simulink model "CDF_VSVPWM_Grid_Parallel"  (R2024b)
settings: Solver=VariableStepAuto  StartTime=0.0  StopTime=0.1  FixedStep=1e-7  MaxStep=1e-5  RelTol=1e-4
InitFcn:
  | Ts=1/10e3;
  | Udc=600;
  | C=4000e-6;
  | m=0.7;
libraries: simulink x24, spsMosfetLib x24, spsSeriesRLCBranchLib x16, spsDiodeLib x12, spsabctoAlphaBetaZeroLib x5, spsThreePhaseVIMeasurementLib x4, spsVoltageMeasurementLib x4, spsAlphaBetaZerotoabcLib x2, spsStairGeneratorLib x2, spsOnDelayLib x2, spsdq0toAlphaBetaZeroLib x2, spsBreakerLib x1
(part 3 of 5)

== system CDF_VSVPWM_Grid_Parallel/VSVPWM/生成时间调制波/Ⅴ扇区的调制信号计算/1-4 ==
  time : Inport
  Demux : Demux  Outputs=5
  MATLAB Function : MATLAB Function  TreatAsAtomicUnit=on
  MATLAB Function1 : MATLAB Function  TreatAsAtomicUnit=on
  MATLAB Function2 : MATLAB Function  TreatAsAtomicUnit=on
  Mux : Mux  Inputs=6
  Tabc12 : Outport
  wires:
  time/1 -> Demux/1
  Demux/3 -> MATLAB Function2/3(T3), MATLAB Function1/3(unused), MATLAB Function/3(T3)
  Mux/1 -> Tabc12/1
  Demux/1 -> MATLAB Function2/1(unused), MATLAB Function1/1(T1), MATLAB Function/1(T1)
  Demux/2 -> MATLAB Function2/2(T2), MATLAB Function1/2(T2), MATLAB Function/2(T2)
  Demux/4 -> MATLAB Function2/4(T4), MATLAB Function1/4(unused1), MATLAB Function/4(unused)
  Demux/5 -> MATLAB Function2/5(T5), MATLAB Function1/5(T5), MATLAB Function/5(unused1)
  MATLAB Function/1(ta1) -> Mux/5
  MATLAB Function/2(ta2) -> Mux/6
  MATLAB Function1/1(tb1) -> Mux/1
  MATLAB Function1/2(tb2) -> Mux/2
  MATLAB Function2/1(tc1) -> Mux/3
  MATLAB Function2/2(tc2) -> Mux/4

== system CDF_VSVPWM_Grid_Parallel/VSVPWM/生成时间调制波/Ⅴ扇区的调制信号计算/1-5 ==
  time : Inport
  Demux : Demux  Outputs=5
  MATLAB Function : MATLAB Function  TreatAsAtomicUnit=on
  MATLAB Function1 : MATLAB Function  TreatAsAtomicUnit=on
  MATLAB Function2 : MATLAB Function  TreatAsAtomicUnit=on
  Mux : Mux  Inputs=6
  Tabc12 : Outport
  wires:
  Demux/5 -> MATLAB Function/5(unused), MATLAB Function1/5(T5), MATLAB Function2/5(T5)
  Demux/4 -> MATLAB Function/4(T4), MATLAB Function1/4(T4), MATLAB Function2/4(T4)
  Demux/2 -> MATLAB Function/2(T2), MATLAB Function1/2(T2), MATLAB Function2/2(T2)
  Demux/1 -> MATLAB Function/1(T1), MATLAB Function1/1(T1), MATLAB Function2/1(unused)
  Demux/3 -> MATLAB Function/3(T3), MATLAB Function1/3(unused), MATLAB Function2/3(T3)
  time/1 -> Demux/1
  MATLAB Function1/2(tb2) -> Mux/2
  MATLAB Function2/1(tc1) -> Mux/3
  Mux/1 -> Tabc12/1
  MATLAB Function/1(ta1) -> Mux/5
  MATLAB Function2/2(tc2) -> Mux/4
  MATLAB Function/2(ta2) -> Mux/6
  MATLAB Function1/1(tb1) -> Mux/1

== system CDF_VSVPWM_Grid_Parallel/VSVPWM/生成时间调制波/Ⅵ扇区的调制信号计算/2-1 ==
  time : Inport
  Demux : Demux  Outputs=5
  MATLAB Function : MATLAB Function  TreatAsAtomicUnit=on
  MATLAB Function1 : MATLAB Function  TreatAsAtomicUnit=on
  MATLAB Function2 : MATLAB Function  TreatAsAtomicUnit=on
  Mux : Mux  Inputs=6
  Tabc12 : Outport
  wires:
  time/1 -> Demux/1
  Demux/3 -> MATLAB Function2/3(unused2), MATLAB Function1/3(unused), MATLAB Function/3(unused1)
  Demux/1 -> MATLAB Function2/1(unused), MATLAB Function1/1(T1), MATLAB Function/1(T1)
  Demux/2 -> MATLAB Function2/2(unused1), MATLAB Function1/2(T2), MATLAB Function/2(unused)
  Demux/4 -> MATLAB Function2/4(T4), MATLAB Function1/4(unused1), MATLAB Function/4(unused2)
  Demux/5 -> MATLAB Function2/5(T5), MATLAB Function1/5(unused2), MATLAB Function/5(T5)
  MATLAB Function1/2(tb2) -> Mux/2
  MATLAB Function1/1(tb1) -> Mux/1
  MATLAB Function2/2(tc2) -> Mux/4
  MATLAB Function2/1(tc1) -> Mux/3
  MATLAB Function/2(ta2) -> Mux/6
  MATLAB Function/1(ta1) -> Mux/5
  Mux/1 -> Tabc12/1

== system CDF_VSVPWM_Grid_Parallel/VSVPWM/生成时间调制波/Ⅵ扇区的调制信号计算/2-2 ==
  time : Inport
  Demux : Demux  Outputs=5
  MATLAB Function : MATLAB Function  TreatAsAtomicUnit=on
  MATLAB Function1 : MATLAB Function  TreatAsAtomicUnit=on
  MATLAB Function2 : MATLAB Function  TreatAsAtomicUnit=on
  Mux : Mux  Inputs=6
  Tabc12 : Outport
  wires:
  Demux/5 -> MATLAB Function/5(T5), MATLAB Function1/5(unused1), MATLAB Function2/5(T5)
  Demux/4 -> MATLAB Function/4(unused2), MATLAB Function1/4(unused), MATLAB Function2/4(T4)
  Demux/2 -> MATLAB Function/2(unused), MATLAB Function1/2(T2), MATLAB Function2/2(unused1)
  Demux/1 -> MATLAB Function/1(T1), MATLAB Function1/1(T1), MATLAB Function2/1(unused)
  Demux/3 -> MATLAB Function/3(unused1), MATLAB Function1/3(T3), MATLAB Function2/3(T3)
  time/1 -> Demux/1
  MATLAB Function1/2(tb2) -> Mux/2
  MATLAB Function1/1(tb1) -> Mux/1
  MATLAB Function2/2(tc2) -> Mux/4
  MATLAB Function2/1(tc1) -> Mux/3
  MATLAB Function/2(ta2) -> Mux/6
  MATLAB Function/1(ta1) -> Mux/5
  Mux/1 -> Tabc12/1

== system CDF_VSVPWM_Grid_Parallel/VSVPWM/生成时间调制波/Ⅵ扇区的调制信号计算/2-3 ==
  time : Inport
  Demux : Demux  Outputs=5
  MATLAB Function : MATLAB Function  TreatAsAtomicUnit=on
  MATLAB Function1 : MATLAB Function  TreatAsAtomicUnit=on
  MATLAB Function2 : MATLAB Function  TreatAsAtomicUnit=on
  Mux : Mux  Inputs=6
  Tabc12 : Outport
  wires:
  time/1 -> Demux/1
  Demux/3 -> MATLAB Function2/3(T3), MATLAB Function1/3(T3), MATLAB Function/3(unused)
  Demux/1 -> MATLAB Function2/1(unused), MATLAB Function1/1(T1), MATLAB Function/1(T1)
  Demux/2 -> MATLAB Function2/2(T2), MATLAB Function1/2(T2), MATLAB Function/2(T2)
  Demux/4 -> MATLAB Function2/4(T4), MATLAB Function1/4(unused), MATLAB Function/4(unused1)
  Demux/5 -> MATLAB Function2/5(T5), MATLAB Function1/5(unused1), MATLAB Function/5(T5)
  MATLAB Function1/2(tb2) -> Mux/2
  MATLAB Function1/1(tb1) -> Mux/1
  MATLAB Function2/2(tc2) -> Mux/4
  MATLAB Function2/1(tc1) -> Mux/3
  MATLAB Function/2(ta2) -> Mux/6
  MATLAB Function/1(ta1) -> Mux/5
  Mux/1 -> Tabc12/1

== system CDF_VSVPWM_Grid_Parallel/VSVPWM/生成时间调制波/Ⅵ扇区的调制信号计算/2-4 ==
  time : Inport
  Demux : Demux  Outputs=5
  MATLAB Function : MATLAB Function  TreatAsAtomicUnit=on
  MATLAB Function1 : MATLAB Function  TreatAsAtomicUnit=on
  MATLAB Function2 : MATLAB Function  TreatAsAtomicUnit=on
  Mux : Mux  Inputs=6
  Tabc12 : Outport
  wires:
  Demux/5 -> MATLAB Function/5(T5), MATLAB Function1/5(unused), MATLAB Function2/5(T5)
  Demux/4 -> MATLAB Function/4(T4), MATLAB Function1/4(T4), MATLAB Function2/4(T4)
  Demux/2 -> MATLAB Function/2(unused), MATLAB Function1/2(T2), MATLAB Function2/2(unused1)
  Demux/1 -> MATLAB Function/1(T1), MATLAB Function1/1(T1), MATLAB Function2/1(unused)
  Demux/3 -> MATLAB Function/3(unused1), MATLAB Function1/3(T3), MATLAB Function2/3(T3)
  time/1 -> Demux/1
  MATLAB Function1/2(tb2) -> Mux/2
  MATLAB Function1/1(tb1) -> Mux/1
  MATLAB Function2/2(tc2) -> Mux/4
  MATLAB Function2/1(tc1) -> Mux/3
  MATLAB Function/2(ta2) -> Mux/6
  MATLAB Function/1(ta1) -> Mux/5
  Mux/1 -> Tabc12/1

== system CDF_VSVPWM_Grid_Parallel/VSVPWM/生成时间调制波/Ⅵ扇区的调制信号计算/2-5 ==
  time : Inport
  Demux : Demux  Outputs=5
  MATLAB Function : MATLAB Function  TreatAsAtomicUnit=on
  MATLAB Function1 : MATLAB Function  TreatAsAtomicUnit=on
  MATLAB Function2 : MATLAB Function  TreatAsAtomicUnit=on
  Mux : Mux  Inputs=6
  Tabc12 : Outport
  wires:
  time/1 -> Demux/1
  Demux/3 -> MATLAB Function2/3(T3), MATLAB Function1/3(T3), MATLAB Function/3(unused)
  Demux/1 -> MATLAB Function2/1(unused), MATLAB Function1/1(T1), MATLAB Function/1(T1)
  Demux/2 -> MATLAB Function2/2(T2), MATLAB Function1/2(T2), MATLAB Function/2(T2)
  Demux/4 -> MATLAB Function2/4(T4), MATLAB Function1/4(T4), MATLAB Function/4(T4)
  Demux/5 -> MATLAB Function2/5(T5), MATLAB Function1/5(unused), MATLAB Function/5(T5)
  MATLAB Function1/2(tb2) -> Mux/2
  MATLAB Function1/1(tb1) -> Mux/1
  MATLAB Function2/2(tc2) -> Mux/4
  MATLAB Function2/1(tc1) -> Mux/3
  MATLAB Function/2(ta2) -> Mux/6
  MATLAB Function/1(ta1) -> Mux/5
  Mux/1 -> Tabc12/1

== system CDF_VSVPWM_Grid_Parallel/VSVPWM1/生成时间调制波/Ⅰ扇区的调制信号计算/1-1 ==
  time : Inport
  Demux : Demux  Outputs=5
  MATLAB Function : MATLAB Function  TreatAsAtomicUnit=on
  MATLAB Function1 : MATLAB Function  TreatAsAtomicUnit=on
  MATLAB Function2 : MATLAB Function  TreatAsAtomicUnit=on
  Mux : Mux  Inputs=6
  Scope : Scope
  Tabc12 : Outport
  wires:
  MATLAB Function/1(ta1) -> Mux/1
  time/1 -> Demux/1
  Mux/1 -> Scope/1, Tabc12/1
  Demux/1 -> MATLAB Function2/1(unused), MATLAB Function1/1(T1), MATLAB Function/1(T1)
  MATLAB Function/2(ta2) -> Mux/2
  MATLAB Function1/1(tb1) -> Mux/3
  MATLAB Function1/2(tb2) -> Mux/4
  MATLAB Function2/1(tc1) -> Mux/5
  MATLAB Function2/2(tc2) -> Mux/6
  Demux/2 -> MATLAB Function2/2(unused1), MATLAB Function1/2(unused), MATLAB Function/2(T2)
  Demux/3 -> MATLAB Function2/3(unused2), MATLAB Function1/3(unused1), MATLAB Function/3(unused)
  Demux/4 -> MATLAB Function2/4(T4), MATLAB Function1/4(unused2), MATLAB Function/4(unused1)
  Demux/5 -> MATLAB Function2/5(T5), MATLAB Function1/5(T5), MATLAB Function/5(unused2)

== system CDF_VSVPWM_Grid_Parallel/VSVPWM1/生成时间调制波/Ⅰ扇区的调制信号计算/1-2 ==
  time : Inport
  Demux : Demux  Outputs=5
  MATLAB Function : MATLAB Function  TreatAsAtomicUnit=on
  MATLAB Function1 : MATLAB Function  TreatAsAtomicUnit=on
  MATLAB Function2 : MATLAB Function  TreatAsAtomicUnit=on
  Mux : Mux  Inputs=6
  Tabc12 : Outport
  wires:
  MATLAB Function2/2(tc2) -> Mux/6
  MATLAB Function2/1(tc1) -> Mux/5
  MATLAB Function1/2(tb2) -> Mux/4
  MATLAB Function1/1(tb1) -> Mux/3
  MATLAB Function/2(ta2) -> Mux/2
  Demux/5 -> MATLAB Function/5(unused1), MATLAB Function1/5(T5), MATLAB Function2/5(T5)
  Demux/4 -> MATLAB Function/4(unused), MATLAB Function1/4(unused2), MATLAB Function2/4(T4)
  Demux/2 -> MATLAB Function/2(T2), MATLAB Function1/2(unused), MATLAB Function2/2(unused1)
  Demux/1 -> MATLAB Function/1(T1), MATLAB Function1/1(T1), MATLAB Function2/1(unused)
  Mux/1 -> Tabc12/1
  Demux/3 -> MATLAB Function/3(T3), MATLAB Function1/3(unused1), MATLAB Function2/3(T3)
  time/1 -> Demux/1
  MATLAB Function/1(ta1) -> Mux/1

== system CDF_VSVPWM_Grid_Parallel/VSVPWM1/生成时间调制波/Ⅰ扇区的调制信号计算/1-3 ==
  time : Inport
  Demux : Demux  Outputs=5
  MATLAB Function : MATLAB Function  TreatAsAtomicUnit=on
  MATLAB Function1 : MATLAB Function  TreatAsAtomicUnit=on
  MATLAB Function2 : MATLAB Function  TreatAsAtomicUnit=on
  Mux : Mux  Inputs=6
  Tabc12 : Outport
  wires:
  MATLAB Function/1(ta1) -> Mux/1
  time/1 -> Demux/1
  Demux/3 -> MATLAB Function2/3(T3), MATLAB Function1/3(unused1), MATLAB Function/3(T3)
  Mux/1 -> Tabc12/1
  Demux/1 -> MATLAB Function2/1(unused), MATLAB Function1/1(T1), MATLAB Function/1(T1)
  Demux/2 -> MATLAB Function2/2(unused1), MATLAB Function1/2(unused), MATLAB Function/2(T2)
  Demux/4 -> MATLAB Function2/4(T4), MATLAB Function1/4(T4), MATLAB Function/4(T4)
  Demux/5 -> MATLAB Function2/5(T5), MATLAB Function1/5(T5), MATLAB Function/5(unused)
  MATLAB Function/2(ta2) -> Mux/2
  MATLAB Function1/1(tb1) -> Mux/3
  MATLAB Function1/2(tb2) -> Mux/4
  MATLAB Function2/1(tc1) -> Mux/5
  MATLAB Function2/2(tc2) -> Mux/6

== system CDF_VSVPWM_Grid_Parallel/VSVPWM1/生成时间调制波/Ⅰ扇区的调制信号计算/1-4 ==
  time : Inport
  Demux : Demux  Outputs=5
  MATLAB Function : MATLAB Function  TreatAsAtomicUnit=on
  MATLAB Function1 : MATLAB Function  TreatAsAtomicUnit=on
  MATLAB Function2 : MATLAB Function  TreatAsAtomicUnit=on
  Mux : Mux  Inputs=6
  Tabc12 : Outport
  wires:
  MATLAB Function2/2(tc2) -> Mux/6
  MATLAB Function2/1(tc1) -> Mux/5
  MATLAB Function1/2(tb2) -> Mux/4
  MATLAB Function1/1(tb1) -> Mux/3
  MATLAB Function/2(ta2) -> Mux/2
  Demux/5 -> MATLAB Function/5(unused1), MATLAB Function1/5(T5), MATLAB Function2/5(T5)
  Demux/4 -> MATLAB Function/4(unused), MATLAB Function1/4(unused1), MATLAB Function2/4(T4)
  Demux/2 -> MATLAB Function/2(T2), MATLAB Function1/2(T2), MATLAB Function2/2(T2)
  Demux/1 -> MATLAB Function/1(T1), MATLAB Function1/1(T1), MATLAB Function2/1(unused)
  Mux/1 -> Tabc12/1
  Demux/3 -> MATLAB Function/3(T3), MATLAB Function1/3(unused), MATLAB Function2/3(T3)
  time/1 -> Demux/1
  MATLAB Function/1(ta1) -> Mux/1

== system CDF_VSVPWM_Grid_Parallel/VSVPWM1/生成时间调制波/Ⅰ扇区的调制信号计算/1-5 ==
  time : Inport
  Demux : Demux  Outputs=5
  MATLAB Function : MATLAB Function  TreatAsAtomicUnit=on
  MATLAB Function1 : MATLAB Function  TreatAsAtomicUnit=on
  MATLAB Function2 : MATLAB Function  TreatAsAtomicUnit=on
  Mux : Mux  Inputs=6
  Tabc12 : Outport
  wires:
  MATLAB Function/1(ta1) -> Mux/1
  time/1 -> Demux/1
  Demux/3 -> MATLAB Function2/3(T3), MATLAB Function1/3(unused), MATLAB Function/3(T3)
  Mux/1 -> Tabc12/1
  Demux/1 -> MATLAB Function2/1(unused), MATLAB Function1/1(T1), MATLAB Function/1(T1)
  Demux/2 -> MATLAB Function2/2(T2), MATLAB Function1/2(T2), MATLAB Function/2(T2)
  Demux/4 -> MATLAB Function2/4(T4), MATLAB Function1/4(T4), MATLAB Function/4(T4)
  Demux/5 -> MATLAB Function2/5(T5), MATLAB Function1/5(T5), MATLAB Function/5(unused)
  MATLAB Function/2(ta2) -> Mux/2
  MATLAB Function1/1(tb1) -> Mux/3
  MATLAB Function1/2(tb2) -> Mux/4
  MATLAB Function2/1(tc1) -> Mux/5
  MATLAB Function2/2(tc2) -> Mux/6

== system CDF_VSVPWM_Grid_Parallel/VSVPWM1/生成时间调制波/Ⅱ扇区的调制信号计算/2-1 ==
  time : Inport
  Demux : Demux  Outputs=5
  MATLAB Function : MATLAB Function  TreatAsAtomicUnit=on
  MATLAB Function1 : MATLAB Function  TreatAsAtomicUnit=on
  MATLAB Function2 : MATLAB Function  TreatAsAtomicUnit=on
  Mux : Mux  Inputs=6
  Scope : Scope  NumInputPorts=6
  Scope1 : Scope
  Tabc12 : Outport
  wires:
  MATLAB Function2/2(tc2) -> Scope/6, Mux/6
  MATLAB Function2/1(tc1) -> Scope/5, Mux/5
  MATLAB Function1/2(tb2) -> Scope/4, Mux/4
  MATLAB Function1/1(tb1) -> Scope/3, Mux/3
  MATLAB Function/2(ta2) -> Mux/2, Scope/2
  Demux/5 -> MATLAB Function/5(T5), MATLAB Function1/5(unused2), MATLAB Function2/5(T5)
  Demux/4 -> MATLAB Function/4(unused2), MATLAB Function1/4(unused1), MATLAB Function2/4(T4)
  Demux/2 -> MATLAB Function/2(unused), MATLAB Function1/2(T2), MATLAB Function2/2(unused1)
  Demux/1 -> MATLAB Function/1(T1), MATLAB Function1/1(T1), MATLAB Function2/1(unused)
  Mux/1 -> Scope1/1, Tabc12/1
  Demux/3 -> MATLAB Function/3(unused1), MATLAB Function1/3(unused), MATLAB Function2/3(unused2)
  time/1 -> Demux/1
  MATLAB Function/1(ta1) -> Scope/1, Mux/1

== system CDF_VSVPWM_Grid_Parallel/VSVPWM1/生成时间调制波/Ⅱ扇区的调制信号计算/2-2 ==
  time : Inport
  Demux : Demux  Outputs=5
  MATLAB Function : MATLAB Function  TreatAsAtomicUnit=on
  MATLAB Function1 : MATLAB Function  TreatAsAtomicUnit=on
  MATLAB Function2 : MATLAB Function  TreatAsAtomicUnit=on
  Mux : Mux  Inputs=6
  Tabc12 : Outport
  wires:
  MATLAB Function/1(ta1) -> Mux/1
  time/1 -> Demux/1
  Demux/3 -> MATLAB Function2/3(T3), MATLAB Function1/3(T3), MATLAB Function/3(unused1)
  Mux/1 -> Tabc12/1
  Demux/1 -> MATLAB Function2/1(unused), MATLAB Function1/1(T1), MATLAB Function/1(T1)
  Demux/2 -> MATLAB Function2/2(unused1), MATLAB Function1/2(T2), MATLAB Function/2(unused)
  Demux/4 -> MATLAB Function2/4(T4), MATLAB Function1/4(unused), MATLAB Function/4(unused2)
  Demux/5 -> MATLAB Function2/5(T5), MATLAB Function1/5(unused1), MATLAB Function/5(T5)
  MATLAB Function/2(ta2) -> Mux/2
  MATLAB Function1/1(tb1) -> Mux/3
  MATLAB Function1/2(tb2) -> Mux/4
  MATLAB Function2/1(tc1) -> Mux/5
  MATLAB Function2/2(tc2) -> Mux/6

== system CDF_VSVPWM_Grid_Parallel/VSVPWM1/生成时间调制波/Ⅱ扇区的调制信号计算/2-3 ==
  time : Inport
  Demux : Demux  Outputs=5
  MATLAB Function : MATLAB Function  TreatAsAtomicUnit=on
  MATLAB Function1 : MATLAB Function  TreatAsAtomicUnit=on
  MATLAB Function2 : MATLAB Function  TreatAsAtomicUnit=on
  Mux : Mux  Inputs=6
  Tabc12 : Outport
  wires:
  MATLAB Function2/2(tc2) -> Mux/6
  MATLAB Function2/1(tc1) -> Mux/5
  MATLAB Function1/2(tb2) -> Mux/4
  MATLAB Function1/1(tb1) -> Mux/3
  MATLAB Function/2(ta2) -> Mux/2
  Demux/5 -> MATLAB Function/5(T5), MATLAB Function1/5(unused1), MATLAB Function2/5(T5)
  Demux/4 -> MATLAB Function/4(unused1), MATLAB Function1/4(unused), MATLAB Function2/4(T4)
  Demux/2 -> MATLAB Function/2(T2), MATLAB Function1/2(T2), MATLAB Function2/2(T2)
  Demux/1 -> MATLAB Function/1(T1), MATLAB Function1/1(T1), MATLAB Function2/1(unused)
  Mux/1 -> Tabc12/1
  Demux/3 -> MATLAB Function/3(unused), MATLAB Function1/3(T3), MATLAB Function2/3(T3)
  time/1 -> Demux/1
  MATLAB Function/1(ta1) -> Mux/1

== system CDF_VSVPWM_Grid_Parallel/VSVPWM1/生成时间调制波/Ⅱ扇区的调制信号计算/2-4 ==
  time : Inport
  Demux : Demux  Outputs=5
  MATLAB Function : MATLAB Function  TreatAsAtomicUnit=on
  MATLAB Function1 : MATLAB Function  TreatAsAtomicUnit=on
  MATLAB Function2 : MATLAB Function  TreatAsAtomicUnit=on
  Mux : Mux  Inputs=6
  Tabc12 : Outport
  wires:
  MATLAB Function/1(ta1) -> Mux/1
  time/1 -> Demux/1
  Demux/3 -> MATLAB Function2/3(T3), MATLAB Function1/3(T3), MATLAB Function/3(unused1)
  Mux/1 -> Tabc12/1
  Demux/1 -> MATLAB Function2/1(unused), MATLAB Function1/1(T1), MATLAB Function/1(T1)
  Demux/2 -> MATLAB Function2/2(unused1), MATLAB Function1/2(T2), MATLAB Function/2(unused)
  Demux/4 -> MATLAB Function2/4(T4), MATLAB Function1/4(T4), MATLAB Function/4(T4)
  Demux/5 -> MATLAB Function2/5(T5), MATLAB Function1/5(unused), MATLAB Function/5(T5)
  MATLAB Function/2(ta2) -> Mux/2
  MATLAB Function1/1(tb1) -> Mux/3
  MATLAB Function1/2(tb2) -> Mux/4
  MATLAB Function2/1(tc1) -> Mux/5
  MATLAB Function2/2(tc2) -> Mux/6

== system CDF_VSVPWM_Grid_Parallel/VSVPWM1/生成时间调制波/Ⅱ扇区的调制信号计算/2-5 ==
  time : Inport
  Demux : Demux  Outputs=5
  MATLAB Function : MATLAB Function  TreatAsAtomicUnit=on
  MATLAB Function1 : MATLAB Function  TreatAsAtomicUnit=on
  MATLAB Function2 : MATLAB Function  TreatAsAtomicUnit=on
  Mux : Mux  Inputs=6
  Tabc12 : Outport
  wires:
  MATLAB Function2/2(tc2) -> Mux/6
  MATLAB Function2/1(tc1) -> Mux/5
  MATLAB Function1/2(tb2) -> Mux/4
  MATLAB Function1/1(tb1) -> Mux/3
  MATLAB Function/2(ta2) -> Mux/2
  Demux/5 -> MATLAB Function/5(T5), MATLAB Function1/5(unused), MATLAB Function2/5(T5)
  Demux/4 -> MATLAB Function/4(T4), MATLAB Function1/4(T4), MATLAB Function2/4(T4)
  Demux/2 -> MATLAB Function/2(T2), MATLAB Function1/2(T2), MATLAB Function2/2(T2)
  Demux/1 -> MATLAB Function/1(T1), MATLAB Function1/1(T1), MATLAB Function2/1(unused)
  Mux/1 -> Tabc12/1
  Demux/3 -> MATLAB Function/3(unused), MATLAB Function1/3(T3), MATLAB Function2/3(T3)
  time/1 -> Demux/1
  MATLAB Function/1(ta1) -> Mux/1

== system CDF_VSVPWM_Grid_Parallel/VSVPWM1/生成时间调制波/Ⅲ扇区的调制信号计算1/1-1 ==
  time : Inport
  Demux : Demux  Outputs=5
  MATLAB Function : MATLAB Function  TreatAsAtomicUnit=on
  MATLAB Function1 : MATLAB Function  TreatAsAtomicUnit=on
  MATLAB Function2 : MATLAB Function  TreatAsAtomicUnit=on
  Mux : Mux  Inputs=6
  Scope : Scope  NumInputPorts=6
  Scope1 : Scope
  Tabc12 : Outport
  wires:
  Demux/5 -> MATLAB Function/5(unused2), MATLAB Function1/5(T5), MATLAB Function2/5(T5)
  Demux/4 -> MATLAB Function/4(unused1), MATLAB Function1/4(unused2), MATLAB Function2/4(T4)
  Demux/3 -> MATLAB Function/3(unused), MATLAB Function1/3(unused1), MATLAB Function2/3(unused2)
  Demux/2 -> MATLAB Function/2(T2), MATLAB Function1/2(unused), MATLAB Function2/2(unused1)
  Demux/1 -> MATLAB Function/1(T1), MATLAB Function1/1(T1), MATLAB Function2/1(unused)
  Mux/1 -> Scope1/1, Tabc12/1
  time/1 -> Demux/1
  MATLAB Function/1(ta1) -> Scope/1, Mux/3
  MATLAB Function/2(ta2) -> Mux/4, Scope/2
  MATLAB Function1/1(tb1) -> Scope/3, Mux/5
  MATLAB Function1/2(tb2) -> Mux/6, Scope/4
  MATLAB Function2/1(tc1) -> Scope/5, Mux/1
  MATLAB Function2/2(tc2) -> Scope/6, Mux/2

== system CDF_VSVPWM_Grid_Parallel/VSVPWM1/生成时间调制波/Ⅲ扇区的调制信号计算1/1-2 ==
  time : Inport
  Demux : Demux  Outputs=5
  MATLAB Function : MATLAB Function  TreatAsAtomicUnit=on
  MATLAB Function1 : MATLAB Function  TreatAsAtomicUnit=on
  MATLAB Function2 : MATLAB Function  TreatAsAtomicUnit=on
  Mux : Mux  Inputs=6
  Tabc12 : Outport
  wires:
  time/1 -> Demux/1
  Demux/3 -> MATLAB Function2/3(T3), MATLAB Function1/3(unused1), MATLAB Function/3(T3)
  Mux/1 -> Tabc12/1
  Demux/1 -> MATLAB Function2/1(unused), MATLAB Function1/1(T1), MATLAB Function/1(T1)
  Demux/2 -> MATLAB Function2/2(unused1), MATLAB Function1/2(unused), MATLAB Function/2(T2)
  Demux/4 -> MATLAB Function2/4(T4), MATLAB Function1/4(unused2), MATLAB Function/4(unused)
  Demux/5 -> MATLAB Function2/5(T5), MATLAB Function1/5(T5), MATLAB Function/5(unused1)
  MATLAB Function/1(ta1) -> Mux/3
  MATLAB Function/2(ta2) -> Mux/4
  MATLAB Function1/1(tb1) -> Mux/5
  MATLAB Function1/2(tb2) -> Mux/6
  MATLAB Function2/1(tc1) -> Mux/1
  MATLAB Function2/2(tc2) -> Mux/2

== system CDF_VSVPWM_Grid_Parallel/VSVPWM1/生成时间调制波/Ⅲ扇区的调制信号计算1/1-3 ==
  time : Inport
  Demux : Demux  Outputs=5
  MATLAB Function : MATLAB Function  TreatAsAtomicUnit=on
  MATLAB Function1 : MATLAB Function  TreatAsAtomicUnit=on
  MATLAB Function2 : MATLAB Function  TreatAsAtomicUnit=on
  Mux : Mux  Inputs=6
  Scope1 : Scope
  Tabc12 : Outport
  wires:
  Demux/5 -> MATLAB Function/5(unused), MATLAB Function1/5(T5), MATLAB Function2/5(T5)
  Demux/4 -> MATLAB Function/4(T4), MATLAB Function1/4(T4), MATLAB Function2/4(T4)
  Demux/2 -> MATLAB Function/2(T2), MATLAB Function1/2(unused), MATLAB Function2/2(unused1)
  Demux/1 -> MATLAB Function/1(T1), MATLAB Function1/1(T1), MATLAB Function2/1(unused)
  Mux/1 -> Scope1/1, Tabc12/1
  Demux/3 -> MATLAB Function/3(T3), MATLAB Function1/3(unused1), MATLAB Function2/3(T3)
  time/1 -> Demux/1
  MATLAB Function/1(ta1) -> Mux/3
  MATLAB Function/2(ta2) -> Mux/4
  MATLAB Function1/1(tb1) -> Mux/5
  MATLAB Function1/2(tb2) -> Mux/6
  MATLAB Function2/1(tc1) -> Mux/1
  MATLAB Function2/2(tc2) -> Mux/2

== system CDF_VSVPWM_Grid_Parallel/VSVPWM1/生成时间调制波/Ⅲ扇区的调制信号计算1/1-4 ==
  time : Inport
  Demux : Demux  Outputs=5
  MATLAB Function : MATLAB Function  TreatAsAtomicUnit=on
  MATLAB Function1 : MATLAB Function  TreatAsAtomicUnit=on
  MATLAB Function2 : MATLAB Function  TreatAsAtomicUnit=on
  Mux : Mux  Inputs=6
  Tabc12 : Outport
  wires:
  time/1 -> Demux/1
  Demux/3 -> MATLAB Function2/3(T3), MATLAB Function1/3(unused), MATLAB Function/3(T3)
  Mux/1 -> Tabc12/1
  Demux/1 -> MATLAB Function2/1(unused), MATLAB Function1/1(T1), MATLAB Function/1(T1)
  Demux/2 -> MATLAB Function2/2(T2), MATLAB Function1/2(T2), MATLAB Function/2(T2)
  Demux/4 -> MATLAB Function2/4(T4), MATLAB Function1/4(unused1), MATLAB Function/4(unused)
  Demux/5 -> MATLAB Function2/5(T5), MATLAB Function1/5(T5), MATLAB Function/5(unused1)
  MATLAB Function/1(ta1) -> Mux/3
  MATLAB Function/2(ta2) -> Mux/4
  MATLAB Function1/1(tb1) -> Mux/5
  MATLAB Function1/2(tb2) -> Mux/6
  MATLAB Function2/1(tc1) -> Mux/1
  MATLAB Function2/2(tc2) -> Mux/2

== system CDF_VSVPWM_Grid_Parallel/VSVPWM1/生成时间调制波/Ⅲ扇区的调制信号计算1/1-5 ==
  time : Inport
  Demux : Demux  Outputs=5
  MATLAB Function : MATLAB Function  TreatAsAtomicUnit=on
  MATLAB Function1 : MATLAB Function  TreatAsAtomicUnit=on
  MATLAB Function2 : MATLAB Function  TreatAsAtomicUnit=on
  Mux : Mux  Inputs=6
  Tabc12 : Outport
  wires:
  Demux/5 -> MATLAB Function/5(unused), MATLAB Function1/5(T5), MATLAB Function2/5(T5)
  Demux/4 -> MATLAB Function/4(T4), MATLAB Function1/4(T4), MATLAB Function2/4(T4)
  Demux/2 -> MATLAB Function/2(T2), MATLAB Function1/2(T2), MATLAB Function2/2(T2)
  Demux/1 -> MATLAB Function/1(T1), MATLAB Function1/1(T1), MATLAB Function2/1(unused)
  Mux/1 -> Tabc12/1
  Demux/3 -> MATLAB Function/3(T3), MATLAB Function1/3(unused), MATLAB Function2/3(T3)
  time/1 -> Demux/1
  MATLAB Function/1(ta1) -> Mux/3
  MATLAB Function/2(ta2) -> Mux/4
  MATLAB Function1/1(tb1) -> Mux/5
  MATLAB Function1/2(tb2) -> Mux/6
  MATLAB Function2/1(tc1) -> Mux/1
  MATLAB Function2/2(tc2) -> Mux/2

== system CDF_VSVPWM_Grid_Parallel/VSVPWM1/生成时间调制波/Ⅳ扇区的调制信号计算/2-1 ==
  time : Inport
  Demux : Demux  Outputs=5
  MATLAB Function : MATLAB Function  TreatAsAtomicUnit=on
  MATLAB Function1 : MATLAB Function  TreatAsAtomicUnit=on
  MATLAB Function2 : MATLAB Function  TreatAsAtomicUnit=on
  Mux : Mux  Inputs=6
  Scope : Scope
  Tabc12 : Outport
  wires:
  time/1 -> Demux/1
  Demux/3 -> MATLAB Function2/3(unused2), MATLAB Function1/3(unused), MATLAB Function/3(unused1)
  Demux/1 -> MATLAB Function2/1(unused), MATLAB Function1/1(T1), MATLAB Function/1(T1)
  Demux/2 -> MATLAB Function2/2(unused1), MATLAB Function1/2(T2), MATLAB Function/2(unused)
  Demux/4 -> MATLAB Function2/4(T4), MATLAB Function1/4(unused1), MATLAB Function/4(unused2)
  Demux/5 -> MATLAB Function2/5(T5), MATLAB Function1/5(unused2), MATLAB Function/5(T5)
  MATLAB Function/2(ta2) -> Mux/4
  MATLAB Function2/1(tc1) -> Mux/1
  MATLAB Function/1(ta1) -> Mux/3
  MATLAB Function2/2(tc2) -> Mux/2
  MATLAB Function1/2(tb2) -> Mux/6
  Mux/1 -> Scope/1, Tabc12/1
  MATLAB Function1/1(tb1) -> Mux/5
